simulink model "AcasXuClosedLoop_all_comp2017b"  (Simulink 9.0)
settings: Solver=VariableStepAuto  StartTime=0.0  StopTime=20  RelTol=1e-3
libraries: neural x14040, simulink x2
(part 33 of 534)

== system AcasXuClosedLoop_all_comp2017b/networks/Networks_m_8/AcasXu_2_8/Layer 3 ==
  "a{2} " : Inport  PortDimensions=50
      mask Display: e = 0.06;c1 = [120 230 180]/255;c2 = ([120 230 180]+0)/(256+128);c3 = ([120 230 180]+128)/(256+128);patch([0 1 1 0],[0 0 1 1],c1);patch([0 1 1 1-e 1-e 0+e],[0 0 1 1-e 0+e 0+e],c2);patch([0 0 1 1-e 0+e 0+e],[0 1 1 1-e 1-e 0+e],c3);plot([0 1 1 0 0],[0 0 1 1 0]);
  Delays 1 : Subsystem
      mask Display: e = 0.06;c1 = [120 150 230]/255;c2 = ([120 150 230]+0)/383;c3 = ([120 150 230]+128)/383;patch([0 1 1 0],[0 0 1 1],c1);patch([0 1 1 1-e 1-e 0+e],[0 0 1 1-e 0+e 0+e],c2);patch([0 0 1 1-e 0+e 0+e],[0 1 1 1-e 1-e 0+e],c3);plot([0 1 1 0 0],[0 0 1 1 0]);disp('0');
  "LW{3,2}" : Subsystem
      mask Display: e = 0.06;c1 = [120 150 230]/255;c2 = ([120 150 230]+0)/383;c3 = ([120 150 230]+128)/383;patch([0 1 1 0],[0 0 1 1],c1);patch([0 1 1 1-e 1-e 0+e],[0 0 1 1-e 0+e 0+e],c2);patch([0 0 1 1-e 0+e 0+e],[0 1 1 1-e 1-e 0+e],c3);plot([0 1 1 0 0],[0 0 1 1 0]);disp('W');
  "b{3}" : Constant  Value=[0.311346999999999984876097869346267543733119964599609375;-0.04779440000000000077218231808728887699544429779052734375;1.5441700000000000425615098720300011336803436279296875;-3.60245000000000015205614545266143977642059326171875;-2.869829999999999881055146033759228885173797607421875;0.92029199999999999892708046900224871933460235595703125;-0.009370440000000000713153980314018554054200649261474609375;-0.037487100000000002364419771083703381009399890899658203125;-1.995039999999999924540361462277360260486602783203125;1.1536399999999999987920773492078296840190887451171875;-0.27011200000000001875122279670904390513896942138671875;-2.368559999999999998721023075631819665431976318359375;-1.1756699999999999928235183688229881227016448974609375;-0.84421999999999997044142219237983226776123046875;-0.046528800000000002101696594536406337283551692962646484375;-1.7640000000000000124344978758017532527446746826171875;0.263351999999999974999553842280874960124492645263671875;-0.6401289999999999480451151612214744091033935546875;-0.363792999999999977500664272156427614390850067138671875;-0.317437000000000024702018208699882961809635162353515625;-0.728145000000000042206238504149951040744781494140625;2.159330000000000193693949768203310668468475341796875;0.597296000000000049112713895738124847412109375;-1.114549999999999929656269159750081598758697509765625;-0.19800899999999999057109789646347053349018096923828125;-3.28727999999999997982058630441315472126007080078125;1.1621900000000000563460389457759447395801544189453125;1.4158699999999999619149093632586300373077392578125;0.2328610000000000124220633779259514994919300079345703125;-0.0254645000000000010231815394945442676544189453125;0.50952900000000000968469748841016553342342376708984375;1.521290000000000031121771826292388141155242919921875;-2.628670000000000062101435105432756245136260986328125;0.65380400000000005178435458219610154628753662109375;-1.5346699999999999786126636536209844052791595458984375;-0.41622999999999998888000618535443209111690521240234375;-1.4265399999999999192112909440766088664531707763671875;-1.97553000000000000824229573481716215610504150390625;0.7910120000000000484163820146932266652584075927734375;-2.351949999999999985078602549037896096706390380859375;0.48103200000000001512745484433253295719623565673828125;-1.6288000000000000255795384873636066913604736328125;-1.25114999999999998436805981327779591083526611328125;-0.31330299999999999815969431438134051859378814697265625;0.76773700000000000276401124210678972303867340087890625;-0.82172999999999996045829675495042465627193450927734375;-2.05731999999999981554310579667799174785614013671875;0.1407690000000000052349236057125381194055080413818359375;-0.85835799999999995435473465477116405963897705078125;0.99921099999999996033039906251360662281513214111328125]
      mask Display: e = 0.06;c1 = [120 150 230]/255;c2 = ([120 150 230]+0)/383;c3 = ([120 150 230]+128)/383;patch([0 1 1 0],[0 0 1 1],c1);patch([0 1 1 1-e 1-e 0+e],[0 0 1 1-e 0+e 0+e],c2);patch([0 0 1 1-e 0+e 0+e],[0 1 1 1-e 1-e 0+e],c3);plot([0 1 1 0 0],[0 0 1 1 0]);disp('b');
  netsum : Sum  Inputs=2  SaturateOnIntegerOverflow=on  InputSameDT=on  OutDataTypeStr=Inherit: Same as first input
      mask Display: e = 0.06;c1 = [120 150 230]/255;c2 = ([120 150 230]+0)/383;c3 = ([120 150 230]+128)/383;patch([0 1 1 0],[0 0 1 1],c1);patch([0 1 1 1-e 1-e 0+e],[0 0 1 1-e 0+e 0+e],c2);patch([0 0 1 1-e 0+e 0+e],[0 1 1 1-e 1-e 0+e],c3);plot([0 1 1 0 0],[0 0 1 1 0]);disp('+');
  poslin : neural/Transfer Functions/poslin
      settings: SourceProductName=Deep Learning Toolbox  SourceProductBaseCode=NN
  "a{3}" : Outport  InitialOutput=[0;0;0;0;0;0;0;0;0;0;0;0;0;0;0;0;0;0;0;0;0;0;0;0;0;0;0;0;0;0;0;0;0;0;0;0;0;0;0;0;0;0;0;0;0;0;0;0;0;0]
      mask Display: e = 0.06;c1 = [120 230 180]/255;c2 = ([120 230 180]+0)/(256+128);c3 = ([120 230 180]+128)/(256+128);patch([0 1 1 0],[0 0 1 1],c1);patch([0 1 1 1-e 1-e 0+e],[0 0 1 1-e 0+e 0+e],c2);patch([0 0 1 1-e 0+e 0+e],[0 1 1 1-e 1-e 0+e],c3);plot([0 1 1 0 0],[0 0 1 1 0]);
  wires:
  poslin/1 -> "a{3}"/1
  netsum/1 -> poslin/1
  "b{3}"/1 -> netsum/2
  "LW{3,2}"/1("lz{3,2}") -> netsum/1
  Delays 1/1("pd{3,2}") -> "LW{3,2}"/1("ad{3,2}")
  "a{2} "/1 -> Delays 1/1("p{3}")

== system AcasXuClosedLoop_all_comp2017b/networks/Networks_m_8/AcasXu_2_8/Layer 4 ==
  "a{3} " : Inport  PortDimensions=50
      mask Display: e = 0.06;c1 = [120 230 180]/255;c2 = ([120 230 180]+0)/(256+128);c3 = ([120 230 180]+128)/(256+128);patch([0 1 1 0],[0 0 1 1],c1);patch([0 1 1 1-e 1-e 0+e],[0 0 1 1-e 0+e 0+e],c2);patch([0 0 1 1-e 0+e 0+e],[0 1 1 1-e 1-e 0+e],c3);plot([0 1 1 0 0],[0 0 1 1 0]);
  Delays 1 : Subsystem
      mask Display: e = 0.06;c1 = [120 150 230]/255;c2 = ([120 150 230]+0)/383;c3 = ([120 150 230]+128)/383;patch([0 1 1 0],[0 0 1 1],c1);patch([0 1 1 1-e 1-e 0+e],[0 0 1 1-e 0+e 0+e],c2);patch([0 0 1 1-e 0+e 0+e],[0 1 1 1-e 1-e 0+e],c3);plot([0 1 1 0 0],[0 0 1 1 0]);disp('0');
  "LW{4,3}" : Subsystem
      mask Display: e = 0.06;c1 = [120 150 230]/255;c2 = ([120 150 230]+0)/383;c3 = ([120 150 230]+128)/383;patch([0 1 1 0],[0 0 1 1],c1);patch([0 1 1 1-e 1-e 0+e],[0 0 1 1-e 0+e 0+e],c2);patch([0 0 1 1-e 0+e 0+e],[0 1 1 1-e 1-e 0+e],c3);plot([0 1 1 0 0],[0 0 1 1 0]);disp('W');
  "b{4}" : Constant  Value=[-1.02123000000000008213874025386758148670196533203125;1.02827000000000001733724275254644453525543212890625;0.8136710000000000331255023411358706653118133544921875;-0.0381782999999999983486986820935271680355072021484375;3.24415999999999993264054864994250237941741943359375;0.82270399999999999085531499076751060783863067626953125;0.8697850000000000303401748169562779366970062255859375;2.53793999999999986272314345114864408969879150390625;-1.7677799999999999069899558890028856694698333740234375;-1.016880000000000006110667527536861598491668701171875;-2.731970000000000009521272659185342490673065185546875;2.653239999999999820801122041302733123302459716796875;0.17357800000000001006128513836301863193511962890625;-1.030769999999999964046537570538930594921112060546875;-1.8319900000000000073185901783290319144725799560546875;-1.18402999999999991587174008600413799285888671875;1.5293200000000000127187149701057933270931243896484375;1.048059999999999991615595718030817806720733642578125;2.69080999999999992411403582082130014896392822265625;0.80943500000000001559641304993419907987117767333984375;-2.437139999999999862012600715388543903827667236328125;1.566500000000000003552713678800500929355621337890625;-0.007697619999999999636230985089468958904035389423370361328125;-5.392450000000000187583282240666449069976806640625;-0.65350500000000000255795384873636066913604736328125;2.6746900000000000119371179607696831226348876953125;-0.2183569999999999955431206899447715841233730316162109375;-4.08192999999999983629095368087291717529296875;2.040620000000000100470742836478166282176971435546875;1.0368999999999999328537114706705324351787567138671875;-0.593242000000000047066350816749036312103271484375;2.005100000000000104449782156734727323055267333984375;-0.556332999999999966433961162692867219448089599609375;-0.6720070000000000209894324143533594906330108642578125;0.7517650000000000165556457432103343307971954345703125;0.9908989999999999742641421107691712677478790283203125;-0.0199257000000000010497824831645630183629691600799560546875;-0.429881000000000013105960761095047928392887115478515625;0.97181300000000003791456038015894591808319091796875;-3.768260000000000164988023243495263159275054931640625;-1.124610000000000109565689854207448661327362060546875;-0.70215700000000003111466639893478713929653167724609375;3.224060000000000147934997585252858698368072509765625;-2.017959999999999975983655531308613717555999755859375;-0.92493499999999995164756683152518235146999359130859375;1.2576300000000000256505927609396167099475860595703125;-0.91686800000000001631406121305190026760101318359375;2.3874300000000001631406121305190026760101318359375;0.2326959999999999861852728599842521362006664276123046875;-0.38878000000000001445954467271803878247737884521484375]
      mask Display: e = 0.06;c1 = [120 150 230]/255;c2 = ([120 150 230]+0)/383;c3 = ([120 150 230]+128)/383;patch([0 1 1 0],[0 0 1 1],c1);patch([0 1 1 1-e 1-e 0+e],[0 0 1 1-e 0+e 0+e],c2);patch([0 0 1 1-e 0+e 0+e],[0 1 1 1-e 1-e 0+e],c3);plot([0 1 1 0 0],[0 0 1 1 0]);disp('b');
  netsum : Sum  Inputs=2  SaturateOnIntegerOverflow=on  InputSameDT=on  OutDataTypeStr=Inherit: Same as first input
      mask Display: e = 0.06;c1 = [120 150 230]/255;c2 = ([120 150 230]+0)/383;c3 = ([120 150 230]+128)/383;patch([0 1 1 0],[0 0 1 1],c1);patch([0 1 1 1-e 1-e 0+e],[0 0 1 1-e 0+e 0+e],c2);patch([0 0 1 1-e 0+e 0+e],[0 1 1 1-e 1-e 0+e],c3);plot([0 1 1 0 0],[0 0 1 1 0]);disp('+');
  poslin : neural/Transfer Functions/poslin
      settings: SourceProductName=Deep Learning Toolbox  SourceProductBaseCode=NN
  "a{4}" : Outport  InitialOutput=[0;0;0;0;0;0;0;0;0;0;0;0;0;0;0;0;0;0;0;0;0;0;0;0;0;0;0;0;0;0;0;0;0;0;0;0;0;0;0;0;0;0;0;0;0;0;0;0;0;0]
      mask Display: e = 0.06;c1 = [120 230 180]/255;c2 = ([120 230 180]+0)/(256+128);c3 = ([120 230 180]+128)/(256+128);patch([0 1 1 0],[0 0 1 1],c1);patch([0 1 1 1-e 1-e 0+e],[0 0 1 1-e 0+e 0+e],c2);patch([0 0 1 1-e 0+e 0+e],[0 1 1 1-e 1-e 0+e],c3);plot([0 1 1 0 0],[0 0 1 1 0]);
  wires:
  poslin/1 -> "a{4}"/1
  netsum/1 -> poslin/1
  "b{4}"/1 -> netsum/2
  "LW{4,3}"/1("lz{4,3}") -> netsum/1
  Delays 1/1("pd{4,3}") -> "LW{4,3}"/1("ad{4,3}")
  "a{3} "/1 -> Delays 1/1("p{4}")

== system AcasXuClosedLoop_all_comp2017b/networks/Networks_m_8/AcasXu_2_8/Layer 5 ==
  "a{4} " : Inport  PortDimensions=50
      mask Display: e = 0.06;c1 = [120 230 180]/255;c2 = ([120 230 180]+0)/(256+128);c3 = ([120 230 180]+128)/(256+128);patch([0 1 1 0],[0 0 1 1],c1);patch([0 1 1 1-e 1-e 0+e],[0 0 1 1-e 0+e 0+e],c2);patch([0 0 1 1-e 0+e 0+e],[0 1 1 1-e 1-e 0+e],c3);plot([0 1 1 0 0],[0 0 1 1 0]);
  Delays 1 : Subsystem
      mask Display: e = 0.06;c1 = [120 150 230]/255;c2 = ([120 150 230]+0)/383;c3 = ([120 150 230]+128)/383;patch([0 1 1 0],[0 0 1 1],c1);patch([0 1 1 1-e 1-e 0+e],[0 0 1 1-e 0+e 0+e],c2);patch([0 0 1 1-e 0+e 0+e],[0 1 1 1-e 1-e 0+e],c3);plot([0 1 1 0 0],[0 0 1 1 0]);disp('0');
  "LW{5,4}" : Subsystem
      mask Display: e = 0.06;c1 = [120 150 230]/255;c2 = ([120 150 230]+0)/383;c3 = ([120 150 230]+128)/383;patch([0 1 1 0],[0 0 1 1],c1);patch([0 1 1 1-e 1-e 0+e],[0 0 1 1-e 0+e 0+e],c2);patch([0 0 1 1-e 0+e 0+e],[0 1 1 1-e 1-e 0+e],c3);plot([0 1 1 0 0],[0 0 1 1 0]);disp('W');
  "b{5}" : Constant  Value=[0.439529999999999976267872625612653791904449462890625;-3.045990000000000197388771994155831634998321533203125;1.6428799999999998959765434847213327884674072265625;2.134069999999999911466375124291516840457916259765625;-0.85371600000000003038991280845948494970798492431640625;-2.125640000000000195967686522635631263256072998046875;-2.931869999999999976125764078460633754730224609375;-0.163594999999999990425436635632649995386600494384765625;-2.072919999999999873807610129006206989288330078125;-0.1506530000000000091286977976778871379792690277099609375;-1.233680000000000109849906948511488735675811767578125;0.67955799999999999538857764491694979369640350341796875;-0.17547699999999999409538986583356745541095733642578125;-2.69808000000000003382183422218076884746551513671875;1.1800200000000000688515910951537080109119415283203125;-3.83903000000000016456169760203920304775238037109375;-0.6446579999999999532889205511310137808322906494140625;3.362039999999999917434934104676358401775360107421875;3.522159999999999957509544401546008884906768798828125;-2.52289999999999992041921359486877918243408203125;1.0348599999999998910737986079766415059566497802734375;-0.10464800000000000490540941200379165820777416229248046875;-1.1666000000000000813571432445314712822437286376953125;2.54544000000000014694023775518871843814849853515625;1.6450899999999999412381157526397146284580230712890625;-3.863020000000000120365939437760971486568450927734375;-1.6920200000000000795097321315552107989788055419921875;0.94795700000000004958877752869739197194576263427734375;-0.4409680000000000266169308815733529627323150634765625;-2.50403000000000020008883439004421234130859375;1.574289999999999967172925607883371412754058837890625;-2.08933999999999997498889570124447345733642578125;1.802210000000000089670493252924643456935882568359375;-2.3291599999999998971134118619374930858612060546875;-1.8626199999999999423749841298558749258518218994140625;-1.063379999999999991899812812334857881069183349609375;1.4418200000000001015365569401183165609836578369140625;1.1552700000000000191135995919466949999332427978515625;-0.0286314000000000012935430504512623883783817291259765625;-3.334210000000000118092202683328650891780853271484375;-3.35700999999999982748022375744767487049102783203125;-0.64380899999999996463628804121981374919414520263671875;-3.8161100000000001131184035330079495906829833984375;1.2076800000000000867572680363082326948642730712890625;0.468355999999999994543031789362430572509765625;1.1593500000000001026734253173344768583774566650390625;0.2924700000000000077449158197850920259952545166015625;0.10594099999999999350830393041178467683494091033935546875;4.27958999999999978314235704601742327213287353515625;-0.55191599999999996217781017548986710608005523681640625]
      mask Display: e = 0.06;c1 = [120 150 230]/255;c2 = ([120 150 230]+0)/383;c3 = ([120 150 230]+128)/383;patch([0 1 1 0],[0 0 1 1],c1);patch([0 1 1 1-e 1-e 0+e],[0 0 1 1-e 0+e 0+e],c2);patch([0 0 1 1-e 0+e 0+e],[0 1 1 1-e 1-e 0+e],c3);plot([0 1 1 0 0],[0 0 1 1 0]);disp('b');
  netsum : Sum  Inputs=2  SaturateOnIntegerOverflow=on  InputSameDT=on  OutDataTypeStr=Inherit: Same as first input
      mask Display: e = 0.06;c1 = [120 150 230]/255;c2 = ([120 150 230]+0)/383;c3 = ([120 150 230]+128)/383;patch([0 1 1 0],[0 0 1 1],c1);patch([0 1 1 1-e 1-e 0+e],[0 0 1 1-e 0+e 0+e],c2);patch([0 0 1 1-e 0+e 0+e],[0 1 1 1-e 1-e 0+e],c3);plot([0 1 1 0 0],[0 0 1 1 0]);disp('+');
  poslin : neural/Transfer Functions/poslin
      settings: SourceProductName=Deep Learning Toolbox  SourceProductBaseCode=NN
  "a{5}" : Outport  InitialOutput=[0;0;0;0;0;0;0;0;0;0;0;0;0;0;0;0;0;0;0;0;0;0;0;0;0;0;0;0;0;0;0;0;0;0;0;0;0;0;0;0;0;0;0;0;0;0;0;0;0;0]
      mask Display: e = 0.06;c1 = [120 230 180]/255;c2 = ([120 230 180]+0)/(256+128);c3 = ([120 230 180]+128)/(256+128);patch([0 1 1 0],[0 0 1 1],c1);patch([0 1 1 1-e 1-e 0+e],[0 0 1 1-e 0+e 0+e],c2);patch([0 0 1 1-e 0+e 0+e],[0 1 1 1-e 1-e 0+e],c3);plot([0 1 1 0 0],[0 0 1 1 0]);
  wires:
  poslin/1 -> "a{5}"/1
  netsum/1 -> poslin/1
  "b{5}"/1 -> netsum/2
  "LW{5,4}"/1("lz{5,4}") -> netsum/1
  Delays 1/1("pd{5,4}") -> "LW{5,4}"/1("ad{5,4}")
  "a{4} "/1 -> Delays 1/1("p{5}")

== system AcasXuClosedLoop_all_comp2017b/networks/Networks_m_8/AcasXu_2_8/Layer 6 ==
  "a{5} " : Inport  PortDimensions=50
      mask Display: e = 0.06;c1 = [120 230 180]/255;c2 = ([120 230 180]+0)/(256+128);c3 = ([120 230 180]+128)/(256+128);patch([0 1 1 0],[0 0 1 1],c1);patch([0 1 1 1-e 1-e 0+e],[0 0 1 1-e 0+e 0+e],c2);patch([0 0 1 1-e 0+e 0+e],[0 1 1 1-e 1-e 0+e],c3);plot([0 1 1 0 0],[0 0 1 1 0]);
  Delays 1 : Subsystem
      mask Display: e = 0.06;c1 = [120 150 230]/255;c2 = ([120 150 230]+0)/383;c3 = ([120 150 230]+128)/383;patch([0 1 1 0],[0 0 1 1],c1);patch([0 1 1 1-e 1-e 0+e],[0 0 1 1-e 0+e 0+e],c2);patch([0 0 1 1-e 0+e 0+e],[0 1 1 1-e 1-e 0+e],c3);plot([0 1 1 0 0],[0 0 1 1 0]);disp('0');
  "LW{6,5}" : Subsystem
      mask Display: e = 0.06;c1 = [120 150 230]/255;c2 = ([120 150 230]+0)/383;c3 = ([120 150 230]+128)/383;patch([0 1 1 0],[0 0 1 1],c1);patch([0 1 1 1-e 1-e 0+e],[0 0 1 1-e 0+e 0+e],c2);patch([0 0 1 1-e 0+e 0+e],[0 1 1 1-e 1-e 0+e],c3);plot([0 1 1 0 0],[0 0 1 1 0]);disp('W');
  "b{6}" : Constant  Value=[-2.027499999999999857891452847979962825775146484375;4.50964999999999971436182022443972527980804443359375;-3.793930000000000024584778657299466431140899658203125;-0.1535459999999999880504475413545151241123676300048828125;-3.553020000000000067075234255753457546234130859375;1.93480000000000007531752999057061970233917236328125;1.221379999999999910187398199923336505889892578125;1.699780000000000068638428274425677955150604248046875;-0.52099700000000004340705572758452035486698150634765625;1.6300600000000000644462261334410868585109710693359375;-3.224349999999999827338115210295654833316802978515625;1.3425800000000001066524646375910378992557525634765625;0.0228412999999999984102050376577608403749763965606689453125;-1.138949999999999906918901615426875650882720947265625;1.7714000000000000856203996590920723974704742431640625;0.4216779999999999972715158946812152862548828125;-2.5430299999999999016608853708021342754364013671875;0.335123999999999977461584421689622104167938232421875;1.7138899999999999135269490579958073794841766357421875;-0.45187699999999997313437916091061197221279144287109375;-0.9628259999999999596553834635415114462375640869140625;-0.00781589000000000068413275045031696208752691745758056640625;-1.29872999999999994003019310184754431247711181640625;0.0081822200000000004649347573604245553724467754364013671875;2.091959999999999819664253664086572825908660888671875;-1.1268100000000000893152218850445933640003204345703125;3.95655000000000001136868377216160297393798828125;-0.6587199999999999722177790317800827324390411376953125;-0.6610620000000000384687837140518240630626678466796875;-0.038619300000000002237587892750525497831404209136962890625;0.327844999999999997530863993233651854097843170166015625;-1.0096700000000000674305056236335076391696929931640625;0.817630999999999996674659996642731130123138427734375;1.873780000000000001136868377216160297393798828125;-3.407649999999999845812226340058259665966033935546875;1.123839999999999950119899949640966951847076416015625;0.460316000000000002945199639725615270435810089111328125;1.608049999999999979394260662957094609737396240234375;0.482989999999999974900077859274460934102535247802734375;1.625010000000000065512040237081237137317657470703125;1.0257300000000000306243919112603180110454559326171875;-0.52110599999999995812771658165729604661464691162109375;-1.64627999999999996560973158921115100383758544921875;-0.484258000000000021767476710010669194161891937255859375;1.179980000000000028848035071860067546367645263671875;1.634889999999999954383156364201568067073822021484375;-0.021521800000000000652189413585801958106458187103271484375;-0.1870880000000000042970071945092058740556240081787109375;1.3349100000000000409983158533577807247638702392578125;-2.320510000000000072617467594682238996028900146484375]
      mask Display: e = 0.06;c1 = [120 150 230]/255;c2 = ([120 150 230]+0)/383;c3 = ([120 150 230]+128)/383;patch([0 1 1 0],[0 0 1 1],c1);patch([0 1 1 1-e 1-e 0+e],[0 0 1 1-e 0+e 0+e],c2);patch([0 0 1 1-e 0+e 0+e],[0 1 1 1-e 1-e 0+e],c3);plot([0 1 1 0 0],[0 0 1 1 0]);disp('b');
  netsum : Sum  Inputs=2  SaturateOnIntegerOverflow=on  InputSameDT=on  OutDataTypeStr=Inherit: Same as first input
      mask Display: e = 0.06;c1 = [120 150 230]/255;c2 = ([120 150 230]+0)/383;c3 = ([120 150 230]+128)/383;patch([0 1 1 0],[0 0 1 1],c1);patch([0 1 1 1-e 1-e 0+e],[0 0 1 1-e 0+e 0+e],c2);patch([0 0 1 1-e 0+e 0+e],[0 1 1 1-e 1-e 0+e],c3);plot([0 1 1 0 0],[0 0 1 1 0]);disp('+');
  poslin : neural/Transfer Functions/poslin
      settings: SourceProductName=Deep Learning Toolbox  SourceProductBaseCode=NN
  "a{6}" : Outport  InitialOutput=[0;0;0;0;0;0;0;0;0;0;0;0;0;0;0;0;0;0;0;0;0;0;0;0;0;0;0;0;0;0;0;0;0;0;0;0;0;0;0;0;0;0;0;0;0;0;0;0;0;0]
      mask Display: e = 0.06;c1 = [120 230 180]/255;c2 = ([120 230 180]+0)/(256+128);c3 = ([120 230 180]+128)/(256+128);patch([0 1 1 0],[0 0 1 1],c1);patch([0 1 1 1-e 1-e 0+e],[0 0 1 1-e 0+e 0+e],c2);patch([0 0 1 1-e 0+e 0+e],[0 1 1 1-e 1-e 0+e],c3);plot([0 1 1 0 0],[0 0 1 1 0]);
  wires:
  poslin/1 -> "a{6}"/1
  netsum/1 -> poslin/1
  "b{6}"/1 -> netsum/2
  "LW{6,5}"/1("lz{6,5}") -> netsum/1
  Delays 1/1("pd{6,5}") -> "LW{6,5}"/1("ad{6,5}")
  "a{5} "/1 -> Delays 1/1("p{6}")

== system AcasXuClosedLoop_all_comp2017b/networks/Networks_m_8/AcasXu_2_8/Layer 7 ==
  "a{6} " : Inport  PortDimensions=50
      mask Display: e = 0.06;c1 = [120 230 180]/255;c2 = ([120 230 180]+0)/(256+128);c3 = ([120 230 180]+128)/(256+128);patch([0 1 1 0],[0 0 1 1],c1);patch([0 1 1 1-e 1-e 0+e],[0 0 1 1-e 0+e 0+e],c2);patch([0 0 1 1-e 0+e 0+e],[0 1 1 1-e 1-e 0+e],c3);plot([0 1 1 0 0],[0 0 1 1 0]);
  Delays 1 : Subsystem
      mask Display: e = 0.06;c1 = [120 150 230]/255;c2 = ([120 150 230]+0)/383;c3 = ([120 150 230]+128)/383;patch([0 1 1 0],[0 0 1 1],c1);patch([0 1 1 1-e 1-e 0+e],[0 0 1 1-e 0+e 0+e],c2);patch([0 0 1 1-e 0+e 0+e],[0 1 1 1-e 1-e 0+e],c3);plot([0 1 1 0 0],[0 0 1 1 0]);disp('0');
  "LW{7,6}" : Subsystem
      mask Display: e = 0.06;c1 = [120 150 230]/255;c2 = ([120 150 230]+0)/383;c3 = ([120 150 230]+128)/383;patch([0 1 1 0],[0 0 1 1],c1);patch([0 1 1 1-e 1-e 0+e],[0 0 1 1-e 0+e 0+e],c2);patch([0 0 1 1-e 0+e 0+e],[0 1 1 1-e 1-e 0+e],c3);plot([0 1 1 0 0],[0 0 1 1 0]);disp('W');
  "b{7}" : Constant  Value=[-0.0215688999999999984902299132727421238087117671966552734375;-0.0199931999999999991668442334002975258044898509979248046875;0.018122699999999998532462797129483078606426715850830078125;-0.018675799999999999345678958206917741335928440093994140625;0.018248199999999999143529549883169238455593585968017578125]
      mask Display: e = 0.06;c1 = [120 150 230]/255;c2 = ([120 150 230]+0)/383;c3 = ([120 150 230]+128)/383;patch([0 1 1 0],[0 0 1 1],c1);patch([0 1 1 1-e 1-e 0+e],[0 0 1 1-e 0+e 0+e],c2);patch([0 0 1 1-e 0+e 0+e],[0 1 1 1-e 1-e 0+e],c3);plot([0 1 1 0 0],[0 0 1 1 0]);disp('b');
  netsum : Sum  Inputs=2  SaturateOnIntegerOverflow=on  InputSameDT=on  OutDataTypeStr=Inherit: Same as first input
      mask Display: e = 0.06;c1 = [120 150 230]/255;c2 = ([120 150 230]+0)/383;c3 = ([120 150 230]+128)/383;patch([0 1 1 0],[0 0 1 1],c1);patch([0 1 1 1-e 1-e 0+e],[0 0 1 1-e 0+e 0+e],c2);patch([0 0 1 1-e 0+e 0+e],[0 1 1 1-e 1-e 0+e],c3);plot([0 1 1 0 0],[0 0 1 1 0]);disp('+');
  purelin : neural/Transfer Functions/purelin
      settings: SourceProductName=Deep Learning Toolbox
  "a{7}" : Outport  InitialOutput=[0;0;0;0;0]
      mask Display: e = 0.06;c1 = [120 230 180]/255;c2 = ([120 230 180]+0)/(256+128);c3 = ([120 230 180]+128)/(256+128);patch([0 1 1 0],[0 0 1 1],c1);patch([0 1 1 1-e 1-e 0+e],[0 0 1 1-e 0+e 0+e],c2);patch([0 0 1 1-e 0+e 0+e],[0 1 1 1-e 1-e 0+e],c3);plot([0 1 1 0 0],[0 0 1 1 0]);
  wires:
  purelin/1 -> "a{7}"/1
  netsum/1 -> purelin/1
  "b{7}"/1 -> netsum/2
  "LW{7,6}"/1("lz{7,6}") -> netsum/1
  Delays 1/1("pd{7,6}") -> "LW{7,6}"/1("ad{7,6}")
  "a{6} "/1 -> Delays 1/1("p{7}")

== system AcasXuClosedLoop_all_comp2017b/networks/Networks_m_8/AcasXu_2_8/Process Input 1 ==
  x : Inport  PortDimensions=5
      mask Display: e = 0.06;c1 = [120 230 180]/255;c2 = ([120 230 180]+0)/(256+128);c3 = ([120 230 180]+128)/(256+128);patch([0 1 1 0],[0 0 1 1],c1);patch([0 1 1 1-e 1-e 0+e],[0 0 1 1-e 0+e 0+e],c2);patch([0 0 1 1-e 0+e 0+e],[0 1 1 1-e 1-e 0+e],c3);plot([0 1 1 0 0],[0 0 1 1 0]);
  p : Outport  PortDimensions=5
      mask Display: e = 0.06;c1 = [120 230 180]/255;c2 = ([120 230 180]+0)/(256+128);c3 = ([120 230 180]+128)/(256+128);patch([0 1 1 0],[0 0 1 1],c1);patch([0 1 1 1-e 1-e 0+e],[0 0 1 1-e 0+e 0+e],c2);patch([0 0 1 1-e 0+e 0+e],[0 1 1 1-e 1-e 0+e],c3);plot([0 1 1 0 0],[0 0 1 1 0]);
  wires:
  x/1 -> p/1

== system AcasXuClosedLoop_all_comp2017b/networks/Networks_m_8/AcasXu_2_8/Process Output 1 ==
  a : Inport  PortDimensions=5
      mask Display: e = 0.06;c1 = [120 230 180]/255;c2 = ([120 230 180]+0)/(256+128);c3 = ([120 230 180]+128)/(256+128);patch([0 1 1 0],[0 0 1 1],c1);patch([0 1 1 1-e 1-e 0+e],[0 0 1 1-e 0+e 0+e],c2);patch([0 0 1 1-e 0+e 0+e],[0 1 1 1-e 1-e 0+e],c3);plot([0 1 1 0 0],[0 0 1 1 0]);
  y : Outport  PortDimensions=5
      mask Display: e = 0.06;c1 = [120 230 180]/255;c2 = ([120 230 180]+0)/(256+128);c3 = ([120 230 180]+128)/(256+128);patch([0 1 1 0],[0 0 1 1],c1);patch([0 1 1 1-e 1-e 0+e],[0 0 1 1-e 0+e 0+e],c2);patch([0 0 1 1-e 0+e 0+e],[0 1 1 1-e 1-e 0+e],c3);plot([0 1 1 0 0],[0 0 1 1 0]);
  wires:
  a/1 -> y/1

== system AcasXuClosedLoop_all_comp2017b/networks/Networks_m_8/AcasXu_3_8/Layer 1 ==
  "p{1}" : Inport  PortDimensions=5
      mask Display: e = 0.06;c1 = [120 230 180]/255;c2 = ([120 230 180]+0)/(256+128);c3 = ([120 230 180]+128)/(256+128);patch([0 1 1 0],[0 0 1 1],c1);patch([0 1 1 1-e 1-e 0+e],[0 0 1 1-e 0+e 0+e],c2);patch([0 0 1 1-e 0+e 0+e],[0 1 1 1-e 1-e 0+e],c3);plot([0 1 1 0 0],[0 0 1 1 0]);
  Delays 1 : Subsystem
      mask Display: e = 0.06;c1 = [120 150 230]/255;c2 = ([120 150 230]+0)/383;c3 = ([120 150 230]+128)/383;patch([0 1 1 0],[0 0 1 1],c1);patch([0 1 1 1-e 1-e 0+e],[0 0 1 1-e 0+e 0+e],c2);patch([0 0 1 1-e 0+e 0+e],[0 1 1 1-e 1-e 0+e],c3);plot([0 1 1 0 0],[0 0 1 1 0]);disp('0');
  "IW{1,1}" : Subsystem
      mask Display: e = 0.06;c1 = [120 150 230]/255;c2 = ([120 150 230]+0)/383;c3 = ([120 150 230]+128)/383;patch([0 1 1 0],[0 0 1 1],c1);patch([0 1 1 1-e 1-e 0+e],[0 0 1 1-e 0+e 0+e],c2);patch([0 0 1 1-e 0+e 0+e],[0 1 1 1-e 1-e 0+e],c3);plot([0 1 1 0 0],[0 0 1 1 0]);disp('W');
  "b{1}" : Constant  Value=[-0.02550650000000000139355194050949648953974246978759765625;-0.03118769999999999875672784810376469977200031280517578125;-0.0229152999999999995583976897250977344810962677001953125;0.110038999999999997925215211580507457256317138671875;-0.5566649999999999653965687684831209480762481689453125;0.094960400000000000364508423444931395351886749267578125;0.0162601999999999989598986616101683466695249080657958984375;0.1323630000000000084270368461147882044315338134765625;-0.075341299999999999936761696517351083457469940185546875;-0.49006899999999997685762309629353694617748260498046875;-0.048466900000000000259259280710466555319726467132568359375;0.00585046000000000003538058734875448863022029399871826171875;0.106881000000000003780087354243732988834381103515625;0.1118989999999999984670040475975838489830493927001953125;-0.049271799999999997543564944635363644920289516448974609375;-0.38872499999999998721023075631819665431976318359375;0.1859929999999999916671100663734250701963901519775390625;-0.0216021999999999984864995639100015978328883647918701171875;-0.2264369999999999993889332472463138401508331298828125;-0.265351999999999976775910681681125424802303314208984375;0.1257089999999999874180645065280259586870670318603515625;-0.01425230000000000073201444905635071336291730403900146484375;-0.12476700000000000290167889716030913405120372772216796875;-0.32577000000000000401456645704456605017185211181640625;-0.03599159999999999859365829024682170711457729339599609375;0.5606489999999999529478600379661656916141510009765625;0.055329099999999999115463111820645281113684177398681640625;0.10207800000000000206767936106189154088497161865234375;-0.380651000000000017120527218139613978564739227294921875;-0.24709700000000001107736125049996189773082733154296875;-0.01978939999999999865831767920099082402884960174560546875;-0.0245798999999999982779552709644121932797133922576904296875;0.054346999999999999475530643167076050303876399993896484375;-0.39385300000000000864019966684281826019287109375;-0.0304525999999999998080202345818179310299456119537353515625;-0.33276600000000000623145979261607863008975982666015625;0.1511990000000000000657252030578092671930789947509765625;-0.1130379999999999995896615700985421426594257354736328125;0.10969099999999999683897300428725429810583591461181640625;-0.19072400000000000463984406451345421373844146728515625;0.0222763999999999982859488767417133203707635402679443359375;0.02573330000000000061799454442734713666141033172607421875;-0.37724299999999999499777914024889469146728515625;-0.10750500000000000333510996597397024743258953094482421875;0.03703889999999999960156316092252382077276706695556640625;-0.08340240000000000153423940218999632634222507476806640625;-0.56099299999999996391153445074451155960559844970703125;-0.73684000000000005048406137575511820614337921142578125;-0.0035632699999999998922983746041381891700439155101776123046875;0.08532689999999999719282328669578419066965579986572265625]
      mask Display: e = 0.06;c1 = [120 150 230]/255;c2 = ([120 150 230]+0)/383;c3 = ([120 150 230]+128)/383;patch([0 1 1 0],[0 0 1 1],c1);patch([0 1 1 1-e 1-e 0+e],[0 0 1 1-e 0+e 0+e],c2);patch([0 0 1 1-e 0+e 0+e],[0 1 1 1-e 1-e 0+e],c3);plot([0 1 1 0 0],[0 0 1 1 0]);disp('b');
  netsum : Sum  Inputs=2  SaturateOnIntegerOverflow=on  InputSameDT=on  OutDataTypeStr=Inherit: Same as first input
      mask Display: e = 0.06;c1 = [120 150 230]/255;c2 = ([120 150 230]+0)/383;c3 = ([120 150 230]+128)/383;patch([0 1 1 0],[0 0 1 1],c1);patch([0 1 1 1-e 1-e 0+e],[0 0 1 1-e 0+e 0+e],c2);patch([0 0 1 1-e 0+e 0+e],[0 1 1 1-e 1-e 0+e],c3);plot([0 1 1 0 0],[0 0 1 1 0]);disp('+');
  poslin : neural/Transfer Functions/poslin
      settings: SourceProductName=Deep Learning Toolbox  SourceProductBaseCode=NN
  "a{1}" : Outport  InitialOutput=[0;0;0;0;0;0;0;0;0;0;0;0;0;0;0;0;0;0;0;0;0;0;0;0;0;0;0;0;0;0;0;0;0;0;0;0;0;0;0;0;0;0;0;0;0;0;0;0;0;0]
      mask Display: e = 0.06;c1 = [120 230 180]/255;c2 = ([120 230 180]+0)/(256+128);c3 = ([120 230 180]+128)/(256+128);patch([0 1 1 0],[0 0 1 1],c1);patch([0 1 1 1-e 1-e 0+e],[0 0 1 1-e 0+e 0+e],c2);patch([0 0 1 1-e 0+e 0+e],[0 1 1 1-e 1-e 0+e],c3);plot([0 1 1 0 0],[0 0 1 1 0]);
  wires:
  poslin/1 -> "a{1}"/1
  netsum/1 -> poslin/1
  "b{1}"/1 -> netsum/2
  "IW{1,1}"/1("iz{1,1}") -> netsum/1
  Delays 1/1("pd{1,1}") -> "IW{1,1}"/1("pd{1,1}")
  "p{1}"/1 -> Delays 1/1("p{1}")

== system AcasXuClosedLoop_all_comp2017b/networks/Networks_m_8/AcasXu_3_8/Layer 2 ==
  "a{1} " : Inport  PortDimensions=50
      mask Display: e = 0.06;c1 = [120 230 180]/255;c2 = ([120 230 180]+0)/(256+128);c3 = ([120 230 180]+128)/(256+128);patch([0 1 1 0],[0 0 1 1],c1);patch([0 1 1 1-e 1-e 0+e],[0 0 1 1-e 0+e 0+e],c2);patch([0 0 1 1-e 0+e 0+e],[0 1 1 1-e 1-e 0+e],c3);plot([0 1 1 0 0],[0 0 1 1 0]);
  Delays 1 : Subsystem
      mask Display: e = 0.06;c1 = [120 150 230]/255;c2 = ([120 150 230]+0)/383;c3 = ([120 150 230]+128)/383;patch([0 1 1 0],[0 0 1 1],c1);patch([0 1 1 1-e 1-e 0+e],[0 0 1 1-e 0+e 0+e],c2);patch([0 0 1 1-e 0+e 0+e],[0 1 1 1-e 1-e 0+e],c3);plot([0 1 1 0 0],[0 0 1 1 0]);disp('0');
  "LW{2,1}" : Subsystem
      mask Display: e = 0.06;c1 = [120 150 230]/255;c2 = ([120 150 230]+0)/383;c3 = ([120 150 230]+128)/383;patch([0 1 1 0],[0 0 1 1],c1);patch([0 1 1 1-e 1-e 0+e],[0 0 1 1-e 0+e 0+e],c2);patch([0 0 1 1-e 0+e 0+e],[0 1 1 1-e 1-e 0+e],c3);plot([0 1 1 0 0],[0 0 1 1 0]);disp('W');
  "b{2}" : Constant  Value=[0.0766471999999999986652454708746518008410930633544921875;0.27917599999999997972821574876434169709682464599609375;-0.0170428999999999997161381770638399757444858551025390625;1.1401600000000000623145979261607863008975982666015625;0.049766499999999998238298104524801601655781269073486328125;7.1046100000000004062207514810012298767105676233768463134765625e-05;-0.383676999999999990276222661123028956353664398193359375;-0.0169435999999999996334931751107433228753507137298583984375;-0.01274680000000000083815177021051567862741649150848388671875;-0.1331839999999999968327557553493534214794635772705078125;-0.06666819999999999690754037828810396604239940643310546875;-0.019204300000000000536726219024785677902400493621826171875;-0.99082400000000003803535264523816294968128204345703125;1.0538199999999999789679350215010344982147216796875;-0.76423399999999996889954445578041486442089080810546875;-1.1999299999999999410960072054876945912837982177734375;0.8901719999999999632933622706332243978977203369140625;-1.0947400000000000463984406451345421373844146728515625;0.94294800000000000839150970932678319513797760009765625;-0.01413389999999999958879559613933452055789530277252197265625;-0.007060450000000000232713848191679062438197433948516845703125;0.03366510000000000335518279825919307768344879150390625;-0.47364499999999998269828438424156047403812408447265625;-0.603558000000000038909320210223086178302764892578125;0.1106000000000000038635761256955447606742382049560546875;-0.82888499999999998291144720496959052979946136474609375;-1.0128999999999999115374293978675268590450286865234375;-0.1137539999999999940083483807029551826417446136474609375;0.55578899999999997749711155847762711346149444580078125;0.6740150000000000307665004584123380482196807861328125;-0.45669599999999999084820956340990960597991943359375;1.2285299999999998998845285314018838107585906982421875;-0.00627866000000000014369394563118476071394979953765869140625;-0.01832330000000000069793060220035840757191181182861328125;-0.051041200000000001846256481030650320462882518768310546875;0.64456400000000002581401759016443975269794464111328125;-0.94357500000000005258016244624741375446319580078125;0.59192100000000003046096708203549496829509735107421875;1.1933499999999999108268866621074266731739044189453125;0.675645999999999968821384754846803843975067138671875;-0.1278070000000000039364067561109550297260284423828125;-0.0028131599999999998794331101947818751796148717403411865234375;-0.713905000000000011795009413617663085460662841796875;0.1014710000000000056363802514169947244226932525634765625;-0.1883100000000000051603166184577275998890399932861328125;-0.00672708999999999980590192905083313235081732273101806640625;0.6396479999999999943582906780648045241832733154296875;0.203154000000000001247002501258975826203823089599609375;0.3039410000000000167119651450775563716888427734375;-0.0194000999999999999834354724725926644168794155120849609375]
      mask Display: e = 0.06;c1 = [120 150 230]/255;c2 = ([120 150 230]+0)/383;c3 = ([120 150 230]+128)/383;patch([0 1 1 0],[0 0 1 1],c1);patch([0 1 1 1-e 1-e 0+e],[0 0 1 1-e 0+e 0+e],c2);patch([0 0 1 1-e 0+e 0+e],[0 1 1 1-e 1-e 0+e],c3);plot([0 1 1 0 0],[0 0 1 1 0]);disp('b');
  netsum : Sum  Inputs=2  SaturateOnIntegerOverflow=on  InputSameDT=on  OutDataTypeStr=Inherit: Same as first input
      mask Display: e = 0.06;c1 = [120 150 230]/255;c2 = ([120 150 230]+0)/383;c3 = ([120 150 230]+128)/383;patch([0 1 1 0],[0 0 1 1],c1);patch([0 1 1 1-e 1-e 0+e],[0 0 1 1-e 0+e 0+e],c2);patch([0 0 1 1-e 0+e 0+e],[0 1 1 1-e 1-e 0+e],c3);plot([0 1 1 0 0],[0 0 1 1 0]);disp('+');
  poslin : neural/Transfer Functions/poslin
      settings: SourceProductName=Deep Learning Toolbox  SourceProductBaseCode=NN
  "a{2}" : Outport  InitialOutput=[0;0;0;0;0;0;0;0;0;0;0;0;0;0;0;0;0;0;0;0;0;0;0;0;0;0;0;0;0;0;0;0;0;0;0;0;0;0;0;0;0;0;0;0;0;0;0;0;0;0]
      mask Display: e = 0.06;c1 = [120 230 180]/255;c2 = ([120 230 180]+0)/(256+128);c3 = ([120 230 180]+128)/(256+128);patch([0 1 1 0],[0 0 1 1],c1);patch([0 1 1 1-e 1-e 0+e],[0 0 1 1-e 0+e 0+e],c2);patch([0 0 1 1-e 0+e 0+e],[0 1 1 1-e 1-e 0+e],c3);plot([0 1 1 0 0],[0 0 1 1 0]);
  wires:
  poslin/1 -> "a{2}"/1
  netsum/1 -> poslin/1
  "b{2}"/1 -> netsum/2
  "LW{2,1}"/1("lz{2,1}") -> netsum/1
  Delays 1/1("pd{2,1}") -> "LW{2,1}"/1("ad{2,1}")
  "a{1} "/1 -> Delays 1/1("p{2}")

== system AcasXuClosedLoop_all_comp2017b/networks/Networks_m_8/AcasXu_3_8/Layer 3 ==
  "a{2} " : Inport  PortDimensions=50
      mask Display: e = 0.06;c1 = [120 230 180]/255;c2 = ([120 230 180]+0)/(256+128);c3 = ([120 230 180]+128)/(256+128);patch([0 1 1 0],[0 0 1 1],c1);patch([0 1 1 1-e 1-e 0+e],[0 0 1 1-e 0+e 0+e],c2);patch([0 0 1 1-e 0+e 0+e],[0 1 1 1-e 1-e 0+e],c3);plot([0 1 1 0 0],[0 0 1 1 0]);
  Delays 1 : Subsystem
      mask Display: e = 0.06;c1 = [120 150 230]/255;c2 = ([120 150 230]+0)/383;c3 = ([120 150 230]+128)/383;patch([0 1 1 0],[0 0 1 1],c1);patch([0 1 1 1-e 1-e 0+e],[0 0 1 1-e 0+e 0+e],c2);patch([0 0 1 1-e 0+e 0+e],[0 1 1 1-e 1-e 0+e],c3);plot([0 1 1 0 0],[0 0 1 1 0]);disp('0');
  "LW{3,2}" : Subsystem
      mask Display: e = 0.06;c1 = [120 150 230]/255;c2 = ([120 150 230]+0)/383;c3 = ([120 150 230]+128)/383;patch([0 1 1 0],[0 0 1 1],c1);patch([0 1 1 1-e 1-e 0+e],[0 0 1 1-e 0+e 0+e],c2);patch([0 0 1 1-e 0+e 0+e],[0 1 1 1-e 1-e 0+e],c3);plot([0 1 1 0 0],[0 0 1 1 0]);disp('W');
  "b{3}" : Constant  Value=[-0.5067049999999999609912038067704997956752777099609375;-0.1580689999999999872937195277700084261596202850341796875;-0.094890799999999997371702420423389412462711334228515625;-1.139769999999999949835682855336926877498626708984375;-0.485723999999999989096721719761262647807598114013671875;0.78898400000000001863753595898742787539958953857421875;-1.796170000000000044337866711430251598358154296875;-2.569010000000000015774048733874224126338958740234375;-1.338859999999999939035433271783404052257537841796875;-0.149681000000000008487432978654396720230579376220703125;-0.59318400000000004457234581423108465969562530517578125;0.552559000000000022367885321727953851222991943359375;-1.430239999999999955804241835721768438816070556640625;-0.88625600000000004374811624074936844408512115478515625;-2.09769000000000005456968210637569427490234375;-1.0982700000000000795097321315552107989788055419921875;-0.007054839999999999722302135296558844856917858123779296875;-0.7484290000000000109281472759903408586978912353515625;-0.378834999999999977315923160858801566064357757568359375;-0.0754628999999999994230392985627986490726470947265625;-0.06119840000000000002078337502098293043673038482666015625;-0.9801260000000000527364818481146357953548431396484375;0.8681259999999999532604988417006097733974456787109375;-0.7170900000000000051869619710487313568592071533203125;-1.3667499999999999094058011905872263014316558837890625;0.014902300000000000268496336275347857736051082611083984375;-0.6758189999999999475477352461894042789936065673828125;-0.0088044799999999999451372190151232643984258174896240234375;-0.92438900000000001622169065740308724343776702880859375;-0.5808050000000000157029944602982141077518463134765625;-0.97779899999999997373123505894909612834453582763671875;-0.334442999999999990290433515838230960071086883544921875;-0.05245339999999999724966670555659220553934574127197265625;-1.615040000000000031121771826292388141155242919921875;-0.2650959999999999983089082888909615576267242431640625;1.1583300000000000817834688859875313937664031982421875;-2.85022000000000019781509763561189174652099609375;-2.198560000000000069775296651641838252544403076171875;-2.64670000000000005258016244624741375446319580078125;-0.026885300000000000864019966684281826019287109375;-1.4800899999999999057109789646347053349018096923828125;2.450089999999999879065626373630948364734649658203125;-0.328023000000000009013234603116870857775211334228515625;-0.036886200000000000931432708739521331153810024261474609375;-0.9353789999999999604796130370232276618480682373046875;-0.09262479999999999324966637459510820917785167694091796875;-0.87377899999999997238120386100490577518939971923828125;-0.40050499999999999989341858963598497211933135986328125;-0.5589420000000000499085217597894370555877685546875;-2.736920000000000019468870959826745092868804931640625]
      mask Display: e = 0.06;c1 = [120 150 230]/255;c2 = ([120 150 230]+0)/383;c3 = ([120 150 230]+128)/383;patch([0 1 1 0],[0 0 1 1],c1);patch([0 1 1 1-e 1-e 0+e],[0 0 1 1-e 0+e 0+e],c2);patch([0 0 1 1-e 0+e 0+e],[0 1 1 1-e 1-e 0+e],c3);plot([0 1 1 0 0],[0 0 1 1 0]);disp('b');
  netsum : Sum  Inputs=2  SaturateOnIntegerOverflow=on  InputSameDT=on  OutDataTypeStr=Inherit: Same as first input
      mask Display: e = 0.06;c1 = [120 150 230]/255;c2 = ([120 150 230]+0)/383;c3 = ([120 150 230]+128)/383;patch([0 1 1 0],[0 0 1 1],c1);patch([0 1 1 1-e 1-e 0+e],[0 0 1 1-e 0+e 0+e],c2);patch([0 0 1 1-e 0+e 0+e],[0 1 1 1-e 1-e 0+e],c3);plot([0 1 1 0 0],[0 0 1 1 0]);disp('+');
  poslin : neural/Transfer Functions/poslin
      settings: SourceProductName=Deep Learning Toolbox  SourceProductBaseCode=NN
  "a{3}" : Outport  InitialOutput=[0;0;0;0;0;0;0;0;0;0;0;0;0;0;0;0;0;0;0;0;0;0;0;0;0;0;0;0;0;0;0;0;0;0;0;0;0;0;0;0;0;0;0;0;0;0;0;0;0;0]
      mask Display: e = 0.06;c1 = [120 230 180]/255;c2 = ([120 230 180]+0)/(256+128);c3 = ([120 230 180]+128)/(256+128);patch([0 1 1 0],[0 0 1 1],c1);patch([0 1 1 1-e 1-e 0+e],[0 0 1 1-e 0+e 0+e],c2);patch([0 0 1 1-e 0+e 0+e],[0 1 1 1-e 1-e 0+e],c3);plot([0 1 1 0 0],[0 0 1 1 0]);
  wires:
  poslin/1 -> "a{3}"/1
  netsum/1 -> poslin/1
  "b{3}"/1 -> netsum/2
  "LW{3,2}"/1("lz{3,2}") -> netsum/1
  Delays 1/1("pd{3,2}") -> "LW{3,2}"/1("ad{3,2}")
  "a{2} "/1 -> Delays 1/1("p{3}")

== system AcasXuClosedLoop_all_comp2017b/networks/Networks_m_8/AcasXu_3_8/Layer 4 ==
  "a{3} " : Inport  PortDimensions=50
      mask Display: e = 0.06;c1 = [120 230 180]/255;c2 = ([120 230 180]+0)/(256+128);c3 = ([120 230 180]+128)/(256+128);patch([0 1 1 0],[0 0 1 1],c1);patch([0 1 1 1-e 1-e 0+e],[0 0 1 1-e 0+e 0+e],c2);patch([0 0 1 1-e 0+e 0+e],[0 1 1 1-e 1-e 0+e],c3);plot([0 1 1 0 0],[0 0 1 1 0]);
  Delays 1 : Subsystem
      mask Display: e = 0.06;c1 = [120 150 230]/255;c2 = ([120 150 230]+0)/383;c3 = ([120 150 230]+128)/383;patch([0 1 1 0],[0 0 1 1],c1);patch([0 1 1 1-e 1-e 0+e],[0 0 1 1-e 0+e 0+e],c2);patch([0 0 1 1-e 0+e 0+e],[0 1 1 1-e 1-e 0+e],c3);plot([0 1 1 0 0],[0 0 1 1 0]);disp('0');
  "LW{4,3}" : Subsystem
      mask Display: e = 0.06;c1 = [120 150 230]/255;c2 = ([120 150 230]+0)/383;c3 = ([120 150 230]+128)/383;patch([0 1 1 0],[0 0 1 1],c1);patch([0 1 1 1-e 1-e 0+e],[0 0 1 1-e 0+e 0+e],c2);patch([0 0 1 1-e 0+e 0+e],[0 1 1 1-e 1-e 0+e],c3);plot([0 1 1 0 0],[0 0 1 1 0]);disp('W');
  "b{4}" : Constant  Value=[-1.904150000000000009237055564881302416324615478515625;-0.74484300000000003283417981947422958910465240478515625;2.300780000000000047322146201622672379016876220703125;-0.63954999999999995186072965225321240723133087158203125;-2.559159999999999879349843467934988439083099365234375;-1.450530000000000097060137704829685389995574951171875;-0.051892199999999999437960696013760752975940704345703125;-4.0411599999999996413180269883014261722564697265625;-1.65115999999999996106225808034650981426239013671875;0.95014100000000001333688715021708048880100250244140625;-0.414712000000000025057289576579933054745197296142578125;-2.430449999999999999289457264239899814128875732421875;-0.6775569999999999648565562893054448068141937255859375;0.72434100000000001262634441445698030292987823486328125;-0.306171000000000026464164193384931422770023345947265625;-3.777480000000000170956582223880104720592498779296875;0.89807800000000004292388666726765222847461700439453125;0.0254777999999999983649079382530544535256922245025634765625;1.33342999999999989313437254168093204498291015625;0.663243000000000026972202249453403055667877197265625;-1.2540299999999999780442294650129042565822601318359375;0.733549000000000006593836587853729724884033203125;-3.672190000000000065227823142777197062969207763671875;2.80527000000000015234036254696547985076904296875;-1.227700000000000013500311979441903531551361083984375;-3.91945999999999994400923242210410535335540771484375;-1.986169999999999991047161529422737658023834228515625;0.164455999999999991079135952531942166388034820556640625;-0.6992949999999999999289457264239899814128875732421875;-0.6369839999999999946567186270840466022491455078125;-0.71661399999999997323385514391702599823474884033203125;-1.3957900000000000861888338477001525461673736572265625;0.54847699999999999231903302643331699073314666748046875;-1.9451799999999999091215840962831862270832061767578125;0.142896999999999996244781641507870517671108245849609375;-0.97064300000000003354472255523432977497577667236328125;-1.3582099999999999173638798311003483831882476806640625;-1.6838800000000000434141611549421213567256927490234375;-0.592767999999999961602270559524185955524444580078125;1.3199199999999999821653773324214853346347808837890625;1.98479999999999989768184605054557323455810546875;0.91801200000000005019273885409347712993621826171875;0.6479120000000000434425828643725253641605377197265625;-0.5968809999999999948983031572424806654453277587890625;-0.036131999999999997452704292300040833652019500732421875;-2.106320000000000192130755749531090259552001953125;-1.970029999999999947846163195208646357059478759765625;0.051782399999999999262723093806926044635474681854248046875;-0.01437159999999999999975575093458246556110680103302001953125;-0.1847290000000000043112180492244078777730464935302734375]
      mask Display: e = 0.06;c1 = [120 150 230]/255;c2 = ([120 150 230]+0)/383;c3 = ([120 150 230]+128)/383;patch([0 1 1 0],[0 0 1 1],c1);patch([0 1 1 1-e 1-e 0+e],[0 0 1 1-e 0+e 0+e],c2);patch([0 0 1 1-e 0+e 0+e],[0 1 1 1-e 1-e 0+e],c3);plot([0 1 1 0 0],[0 0 1 1 0]);disp('b');
  netsum : Sum  Inputs=2  SaturateOnIntegerOverflow=on  InputSameDT=on  OutDataTypeStr=Inherit: Same as first input
      mask Display: e = 0.06;c1 = [120 150 230]/255;c2 = ([120 150 230]+0)/383;c3 = ([120 150 230]+128)/383;patch([0 1 1 0],[0 0 1 1],c1);patch([0 1 1 1-e 1-e 0+e],[0 0 1 1-e 0+e 0+e],c2);patch([0 0 1 1-e 0+e 0+e],[0 1 1 1-e 1-e 0+e],c3);plot([0 1 1 0 0],[0 0 1 1 0]);disp('+');
  poslin : neural/Transfer Functions/poslin
      settings: SourceProductName=Deep Learning Toolbox  SourceProductBaseCode=NN
  "a{4}" : Outport  InitialOutput=[0;0;0;0;0;0;0;0;0;0;0;0;0;0;0;0;0;0;0;0;0;0;0;0;0;0;0;0;0;0;0;0;0;0;0;0;0;0;0;0;0;0;0;0;0;0;0;0;0;0]
      mask Display: e = 0.06;c1 = [120 230 180]/255;c2 = ([120 230 180]+0)/(256+128);c3 = ([120 230 180]+128)/(256+128);patch([0 1 1 0],[0 0 1 1],c1);patch([0 1 1 1-e 1-e 0+e],[0 0 1 1-e 0+e 0+e],c2);patch([0 0 1 1-e 0+e 0+e],[0 1 1 1-e 1-e 0+e],c3);plot([0 1 1 0 0],[0 0 1 1 0]);
  wires:
  poslin/1 -> "a{4}"/1
  netsum/1 -> poslin/1
  "b{4}"/1 -> netsum/2
  "LW{4,3}"/1("lz{4,3}") -> netsum/1
  Delays 1/1("pd{4,3}") -> "LW{4,3}"/1("ad{4,3}")
  "a{3} "/1 -> Delays 1/1("p{4}")
